simulink model "wlan_phy_rx_pmd"  (Simulink 7.8)
settings: Solver=VariableStepDiscrete  SolverMode=SingleTasking  StartTime=0.0  StopTime=rx_sim_time  RelTol=1e-3
PostLoadFcn:
  | wlan_phy_rx_init
InitFcn:
  | wlan_phy_rx_init
StartFcn:
  | wlan_phy_rx_init
values from neighbouring files (symbols this model uses):
  barker_seq20 = 0.95 * circshift(barker_seq20, [0 4]) ./ max(abs(barker_seq20))    [wlan_phy_rx_init.m]
  bit_scrambler_lfsr_bytes = sum(bit_scrambler_lfsr_bytes .* repmat(2.^[0:7], 127, 1),2)    [wlan_phy_rx_init.m]
  deinterleave_ROM = [deinterleave_ROM interleave_11n_64QAM zeros(1, 512-length(interleave_11n_64QAM))]    [wlan_phy_rx_init.m]
  longCorr_coef_i = [3*real(longCorr_coef)]    [wlan_phy_rx_init.m]
  longCorr_coef_q = [3*imag(longCorr_coef)]    [wlan_phy_rx_init.m]
  MAX_CP_LEN = 32    [wlan_phy_rx_init.m]
  MAX_NCBPS = NCBPS_11n_64QAM    (= 312)    [wlan_phy_rx_init.m]
  MAX_NUM_BYTES = 2^16-1    (= 65535)    [wlan_phy_rx_init.m]
  MAX_NUM_SC = 64    [wlan_phy_rx_init.m]
  MAX_NUM_SYMS = 600    [wlan_phy_rx_init.m]
  NCBPS_11a_BPSK = 48    [wlan_phy_rx_init.m]
  NDBPS_11a_BPSK12 = 24    [wlan_phy_rx_init.m]
  PHY_SIGNAL_MIN_LEN = 14    [wlan_phy_rx_init.m]
  preFFT_sampBuff_numSamps = 4*MAX_NUM_SC    (= 256)    [wlan_phy_rx_init.m]
  rx_sim_sig_samp_time = 8    [wlan_phy_rx_init.m]
  scr_ind_rev = zeros(1,128)    [wlan_phy_rx_init.m]
undefined symbols (not defined in the file or in its folder): gcbh, mcs_rom_11ag, mcs_rom_11n, xlBlockLoadCallback, xlGetNormalizedPeriod, xlipgetpartsetting
libraries: xbsIndex_r4 x3862, simulink x1, xbsEDKLib_r4 x1
(part 7 of 23)

== system wlan_phy_rx_pmd/Sync & Antenna Sel/Antenna Selection ==
  Pkt Det : Inport
  Rx I Vec : Inport  Port=2
  Rx Q Vec : Inport  Port=3
  IQ Valid : Inport  Port=4
  RSSI Vec : Inport  Port=5
  Reset : Inport  Port=6
  Ant Sel Logic : Subsystem
  Bus Selector1 : BusSelector  OutputSignals=RFA_Q,RFB_Q,RFC_Q,RFD_Q
  Bus Selector2 : BusSelector  OutputSignals=RFA_RSSI,RFB_RSSI,RFC_RSSI,RFD_RSSI
  Bus Selector3 : BusSelector  OutputSignals=RFA_I,RFB_I,RFC_I,RFD_I
  Bus Selector4 : BusSelector  OutputSignals=signal1,signal2,signal3,signal4
  Delay : xbsIndex_r4/Delay  rst=off  infoeditControl=Selection of Reset will increase slice count due to use of real FFs and instead of SRLs  en=off  latency=1  dbl_ovrd=off  reg_retiming=off  block_type=delay
  Gateway Out{,1..2,4..6} (6 alike) : xbsIndex_r4/Gateway Out  inherit_from_input=off  hdl_port=off  timing_constraint=None  locs_specified=off  LOCs={}  UseAsDAC=off  DACChannel='1'  block_type=gatewayout
  Logical : xbsIndex_r4/Logical  logical_function=AND  inputs=2  en=off  latency=0  precision=Full  arith_type=Unsigned  n_bits=16  bin_pt=0  align_bp=on  dbl_ovrd=off  block_type=logical
  Mux{1..4} (4 alike) : xbsIndex_r4/Mux  inputs=4  en=off  latency=0  precision=Full  arith_type=Unsigned  n_bits=16  bin_pt=14  quantization=Truncate  overflow=Wrap  dbl_ovrd=off  block_type=mux
  Posedge : Subsystem
  RF A Gains : Subsystem
  RF B Gains : Subsystem
  RF C Gains : Subsystem
  RF D Gains : Subsystem
  RSSI & Gain Capture : Subsystem
  Register3 : xbsIndex_r4/Register  init=0  rst=off  en=on  dbl_ovrd=off  block_type=register
      settings: xl_area=[1 1 0 0 0 0 0]  block_version=VER_STRING_GOES_HERE
  Sel Div : Scope  NumInputPorts=10
      settings: TimeRange=16708  YMin=0~-1~-1~0~-1~-1~-1~-1~-1~-1  YMax=1~1~1~1~1~1~1~1~1~1  SaveName=ScopeData42
  Rx I : Outport
  " Rx Q" : Outport  Port=2
  " IQ Valid" : Outport  Port=3
  RSSI : Outport  Port=4
  tag scope: ANTENNA_SEL=global
  wires:
  RF A Gains/1(G_vec) -> Gateway Out2/1, Ant Sel Logic/1(G A)
  RF B Gains/1(G_vec) -> Gateway Out4/1, Ant Sel Logic/2(G B)
  Rx Q Vec/1 -> Bus Selector1/1
  Reset/1 -> RF B Gains/2(Rst), RF A Gains/2(Rst), RF C Gains/2(Rst), RF D Gains/2(Rst)
  Logical/1 -> Posedge/1(D)
  Pkt Det/1 -> Logical/1, Gateway Out/1
  [AGC_DONE] -> Logical/2, Gateway Out1/1
  Posedge/1(Q) -> RF A Gains/1(Capt), RF B Gains/1(Capt), RF C Gains/1(Capt), Delay/1, RF D Gains/1(Capt)
  Delay/1 -> Register3/2
  Register3/1 -> [ANTENNA_SEL]
  IQ Valid/1 -> Bus Selector4/1
  Bus Selector4/1 -> Mux3/2  "<signal1>"
  Bus Selector4/2 -> Mux3/3  "<signal2>"
  Mux3/1 -> " IQ Valid"/1
  Gateway Out2/1 -> Sel Div/3  "G A"
  Gateway Out1/1 -> Sel Div/2  "AGC Done"
  Gateway Out/1 -> Sel Div/1  "Pkt Det"
  Gateway Out4/1 -> Sel Div/4  "G B"
  RF C Gains/1(G_vec) -> Ant Sel Logic/3(G C), Gateway Out5/1
  RF D Gains/1(G_vec) -> Ant Sel Logic/4(G D), Gateway Out6/1
  Gateway Out5/1 -> Sel Div/5  "G C"
  Gateway Out6/1 -> Sel Div/6  "G D"
  Bus Selector4/3 -> Mux3/4  "<signal3>"
  Bus Selector4/4 -> Mux3/5  "<signal4>"
  Rx I Vec/1 -> Bus Selector3/1
  Bus Selector3/1 -> Mux1/2  "<RFA_I>"
  Bus Selector3/2 -> Mux1/3  "<RFB_I>"
  Bus Selector3/3 -> Mux1/4  "<RFC_I>"
  Bus Selector3/4 -> Mux1/5  "<RFD_I>"
  Bus Selector1/1 -> Mux2/2  "<RFA_Q>"
  Bus Selector1/2 -> Mux2/3  "<RFB_Q>"
  Bus Selector1/3 -> Mux2/4  "<RFC_Q>"
  Bus Selector1/4 -> Mux2/5  "<RFD_Q>"
  RSSI Vec/1 -> Bus Selector2/1, RSSI & Gain Capture/1(RSSI Vec)
  Bus Selector2/1 -> Mux4/2  "<RFA_RSSI>"
  Bus Selector2/2 -> Mux4/3  "<RFB_RSSI>"
  Bus Selector2/3 -> Mux4/4  "<RFC_RSSI>"
  Bus Selector2/4 -> Mux4/5  "<RFD_RSSI>"
  Mux4/1 -> RSSI/1
  Ant Sel Logic/1(Ant Sel) -> Mux3/1, Mux1/1, Mux2/1, Mux4/1, Register3/1
  Mux2/1 -> " Rx Q"/1
  Mux1/1 -> Rx I/1

== system wlan_phy_rx_pmd/Sync & Antenna Sel/Debug  Outputs ==
  note: Pulse stretch the timeout signal
      | so it's visible on debug outputs
      | and in down-sampled CS ILA
  LTS : Inport
  LTS Timeout : Inport  Port=2
  Det OFDM : Inport  Port=3
  Det DSSS : Inport  Port=4
  LTS_SYNC : xbsIndex_r4/Gateway Out  inherit_from_input=off  hdl_port=on  timing_constraint=None  locs_specified=off  LOCs={}  UseAsDAC=off  DACChannel='1'  block_type=gatewayout
  Pulse Stretch : Subsystem
  Pulse Stretch1 : Subsystem
  Register{2..11} (10 alike) : xbsIndex_r4/Register  init=0  rst=off  en=off  dbl_ovrd=off  block_type=register
  Slice2 : xbsIndex_r4/Slice  nbits=1  boolean_output=on  mode=Upper Bit Location + Width  bit1=0  base1=MSB of Input  bit0=0  base0=LSB of Input  dbl_ovrd=off  block_type=slice
  dbg_PKT_DET_DSSS : xbsIndex_r4/Gateway Out  inherit_from_input=off  hdl_port=on  timing_constraint=None  locs_specified=off  LOCs={}  UseAsDAC=off  DACChannel='1'  block_type=gatewayout
  dbg_PKT_DET_OFDM : xbsIndex_r4/Gateway Out  inherit_from_input=off  hdl_port=on  timing_constraint=None  locs_specified=off  LOCs={}  UseAsDAC=off  DACChannel='1'  block_type=gatewayout
  dbg_lts_timeout : xbsIndex_r4/Gateway Out  inherit_from_input=off  hdl_port=on  timing_constraint=None  locs_specified=off  LOCs={}  UseAsDAC=off  DACChannel='1'  block_type=gatewayout
  tag scope: CS_LTS_Timeout=global, CS_Pktdet_DSSS=global, CS_Pktdet_OFDM=global
  wires:
  Register8/1 -> Register9/1
  Register9/1 -> LTS_SYNC/1
  Slice2/1 -> Pulse Stretch/1(P)
  Register2/1 -> Register3/1
  Register3/1 -> dbg_lts_timeout/1
  LTS/1 -> Slice2/1
  Register6/1 -> Register4/1, [CS_Pktdet_OFDM]
  Register7/1 -> dbg_PKT_DET_OFDM/1
  Register4/1 -> Register7/1
  Register10/1 -> Register5/1, [CS_Pktdet_DSSS]
  Register11/1 -> dbg_PKT_DET_DSSS/1
  Register5/1 -> Register11/1
  Det OFDM/1 -> Register6/1
  Det DSSS/1 -> Register10/1
  Pulse Stretch/1(P16) -> Register8/1
  LTS Timeout/1 -> Pulse Stretch1/1(P)
  Pulse Stretch1/1(P16) -> Register2/1, [CS_LTS_Timeout]

== system wlan_phy_rx_pmd/Sync & Antenna Sel/LTS Correlation ==
  rx_I : Inport
  rx_Q : Inport  Port=2
  rx_IQ_valid : Inport  Port=3
  RSSI : Inport  Port=4
  En : Inport  Port=5
  Reset : Inport  Port=6
  Bus Creator : BusCreator  Inputs=2
  Bus Creator1 : BusCreator  Inputs=2
  Concat : xbsIndex_r4/Concat  num_inputs=2  dbl_ovrd=off  block_type=concat
  Correlator : Subsystem
  Gateway Out{1..10} (10 alike) : xbsIndex_r4/Gateway Out  inherit_from_input=off  hdl_port=off  timing_constraint=None  locs_specified=off  LOCs={}  UseAsDAC=off  DACChannel='1'  block_type=gatewayout
  Inverter : xbsIndex_r4/Inverter  en=off  latency=0  dbl_ovrd=off  block_type=inv
      settings: xl_area=[0 0 0 0 0 0 0]  block_version=VER_STRING_GOES_HERE
  Inverter1 : xbsIndex_r4/Inverter  en=off  latency=0  dbl_ovrd=off  block_type=inv
      settings: xl_area=[0 0 0 0 0 0 0]  block_version=VER_STRING_GOES_HERE
  LTS Corr : Scope  NumInputPorts=8
      settings: TimeRange=15000  YMin=-1~-1~0~-1~-1~0~-1~-1  YMax=1~1~15000~1~1~1~1~1  SaveName=ScopeData2
  LTS Corr Timeout : Subsystem
  Logical1 : xbsIndex_r4/Logical  logical_function=AND  inputs=2  en=off  latency=0  precision=Full  arith_type=Unsigned  n_bits=16  bin_pt=0  align_bp=on  dbl_ovrd=off  block_type=logical
  Logical2 : xbsIndex_r4/Logical  logical_function=AND  inputs=3  en=off  latency=0  precision=Full  arith_type=Unsigned  n_bits=16  bin_pt=0  align_bp=on  dbl_ovrd=off  block_type=logical
  Logical3 : xbsIndex_r4/Logical  logical_function=AND  inputs=2  en=off  latency=1  precision=Full  arith_type=Unsigned  n_bits=16  bin_pt=0  align_bp=on  dbl_ovrd=off  block_type=logical
  Posedge3 : Subsystem
  Relational2 : xbsIndex_r4/Relational  mode=a>b  en=off  latency=0  dbl_ovrd=off  block_type=relational
      settings: xl_area=[10 0 0 19 0 0 0]  block_version=VER_STRING_GOES_HERE
  S-R Latch : Subsystem
  S-R Latch1 : Subsystem
  Slice : xbsIndex_r4/Slice  nbits=1  boolean_output=off  mode=Upper Bit Location + Width  bit1=0  base1=MSB of Input  bit0=0  base0=LSB of Input  dbl_ovrd=off  block_type=slice
      settings: infoedit="Extracts a given range of bits from each input sample and presents it at the output.  The output type is ordinarily unsigned with binary point at zero, but can be Boolean when the slice is one bit wide.<P><P>Hardware notes: In hardware this block costs nothing."  block_version=10.1.3
  Slice1 : xbsIndex_r4/Slice  nbits=1  boolean_output=off  mode=Upper Bit Location + Width  bit1=0  base1=MSB of Input  bit0=0  base0=LSB of Input  dbl_ovrd=off  block_type=slice
      settings: infoedit="Extracts a given range of bits from each input sample and presents it at the output.  The output type is ordinarily unsigned with binary point at zero, but can be Boolean when the slice is one bit wide.<P><P>Hardware notes: In hardware this block costs nothing."  block_version=10.1.3
  Threshold Select : Subsystem
  Det : Outport
  Timeout : Outport  Port=2
  tag scope: CS_LTS_Det_1=global, CS_LTS_Det_2=global
  wires:
  Gateway Out2/1 -> LTS Corr/3
  Gateway Out4/1 -> LTS Corr/2
  Logical1/1 -> LTS Corr Timeout/3(LTS 1), Concat/2, [CS_LTS_Det_1], Gateway Out8/1
  Inverter/1 -> Logical1/2
  Reset/1 -> S-R Latch/2(R), S-R Latch1/2(R), Gateway Out10/1
  Logical2/1 -> S-R Latch1/1(S), LTS Corr Timeout/4(LTS 2), Concat/1, [CS_LTS_Det_2], Gateway Out9/1
  S-R Latch/1(Q) -> Inverter/1, Logical2/3
  Gateway Out1/1 -> LTS Corr/4
  Gateway Out3/1 -> LTS Corr/5
  rx_I/1 -> Slice/1, Gateway Out4/1
  rx_Q/1 -> Slice1/1, Gateway Out2/1
  Correlator/1(R) -> Relational2/1, Gateway Out1/1
  Gateway Out7/1 -> LTS Corr/8
  Posedge3/1(Q) -> Logical1/1, Logical2/2, S-R Latch/1(S), Gateway Out3/1
  Logical3/1 -> Posedge3/1(D)
  Relational2/1 -> Logical3/2
  En/1 -> LTS Corr Timeout/1(Pkt Det), Logical3/1, Gateway Out6/1, Threshold Select/1(Pkt Det), Gateway Out5/1
  S-R Latch1/1(Q) -> Inverter1/1
  Inverter1/1 -> Logical2/1
  LTS Corr Timeout/1(Timeout) -> Timeout/1, Gateway Out7/1
  Slice/1 -> Correlator/1(Rx I)
  Slice1/1 -> Correlator/2(Rx Q)
  rx_IQ_valid/1 -> LTS Corr Timeout/2(IQ Valid), Correlator/3(rx_IQ_valid)
  Bus Creator/1 -> LTS Corr/6
  Bus Creator1/1 -> LTS Corr/7
  Gateway Out8/1 -> Bus Creator/1
  Gateway Out9/1 -> Bus Creator/2
  Gateway Out6/1 -> Bus Creator1/1
  Gateway Out10/1 -> Bus Creator1/2
  Threshold Select/1(Corr Thresh) -> Relational2/2
  RSSI/1 -> Threshold Select/2(RSSI)
  Concat/1 -> Det/1
  Gateway Out5/1 -> LTS Corr/1

== system wlan_phy_rx_pmd/Sync & Antenna Sel/PHY CCA ==
  LTS Sync : Inport
  LTS Timeout : Inport  Port=2
  IQ Valid : Inport  Port=3
  Det OFDM : Inport  Port=4
  Avg RSSI Vec : Inport  Port=5
  Reset OFDM : Inport  Port=6
  Det DSSS : Inport  Port=7
  Reset DSSS : Inport  Port=8
  Bus Selector1 : BusSelector  OutputSignals=RFA_RSSI,RFB_RSSI,RFC_RSSI,RFD_RSSI
  Gateway Out1 : xbsIndex_r4/Gateway Out  inherit_from_input=off  hdl_port=off  timing_constraint=None  locs_specified=off  LOCs={}  UseAsDAC=off  DACChannel='1'  block_type=gatewayout
  Gateway Out2 : xbsIndex_r4/Gateway Out  inherit_from_input=off  hdl_port=off  timing_constraint=None  locs_specified=off  LOCs={}  UseAsDAC=off  DACChannel='1'  block_type=gatewayout
  Gateway Out4 : xbsIndex_r4/Gateway Out  inherit_from_input=off  hdl_port=off  timing_constraint=None  locs_specified=off  LOCs={}  UseAsDAC=off  DACChannel='1'  block_type=gatewayout
  Logical1 : xbsIndex_r4/Logical  logical_function=OR  inputs=2  en=off  latency=0  precision=Full  arith_type=Unsigned  n_bits=16  bin_pt=0  align_bp=on  dbl_ovrd=off  block_type=logical
  Logical2 : xbsIndex_r4/Logical  logical_function=OR  inputs=4  en=off  latency=0  precision=Full  arith_type=Unsigned  n_bits=16  bin_pt=0  align_bp=on  dbl_ovrd=off  block_type=logical
  Logical3 : xbsIndex_r4/Logical  logical_function=AND  inputs=2  en=off  latency=0  precision=Full  arith_type=Unsigned  n_bits=16  bin_pt=0  align_bp=on  dbl_ovrd=off  block_type=logical
  Logical4 : xbsIndex_r4/Logical  logical_function=AND  inputs=2  en=off  latency=0  precision=Full  arith_type=Unsigned  n_bits=16  bin_pt=0  align_bp=on  dbl_ovrd=off  block_type=logical
  PHY CCA : Scope  NumInputPorts=7
      settings: TimeRange=10000  YMin=-1~-1~0.95~-70~0~0~-0.25  YMax=1~1~1.05~80~1~60000~0.3  SaveName=ScopeData31
  PHY CCA Logic : Subsystem
  Rate*Length BUSY : Subsystem
  Relational{,1..3} (4 alike) : xbsIndex_r4/Relational  mode=a>b  en=off  latency=0  dbl_ovrd=off  block_type=relational
  S-R Latch1 : Subsystem
  S-R Latch2 : Subsystem
  S-R Latch3 : Subsystem
  Slice2 : xbsIndex_r4/Slice  nbits=1  boolean_output=on  mode=Upper Bit Location + Width  bit1=0  base1=MSB of Input  bit0=0  base0=LSB of Input  dbl_ovrd=off  block_type=slice
  tag scope: RX_CCA_BUSY=global, RX_END_OFDM_LAST_SAMP=global, RX_PHY_RATE_LENGTH_ACTIVE=global
  wires:
  [regRx_RSSI_CS_THRESH] -> Relational/2, Relational1/2, Relational2/2, Relational3/2
  Bus Selector1/1 -> Relational/1  "<RFA_RSSI>"
  Logical2/1 -> [RX_CCA_BUSY], Gateway Out2/1  "CCA_BUSY"
  Det OFDM/1 -> S-R Latch2/1(S)
  Logical1/1 -> S-R Latch2/2(R)
  Reset OFDM/1 -> Logical1/1
  LTS Timeout/1 -> Logical1/2
  S-R Latch2/1(Q) -> Logical3/2, Logical2/3
  IQ Valid/1 -> Rate*Length BUSY/1(IQ Valid)
  Logical3/1 -> Rate*Length BUSY/2(Start), Gateway Out4/1
  LTS Sync/1 -> Slice2/1
  Avg RSSI Vec/1 -> Bus Selector1/1
  Slice2/1 -> Logical3/1
  Relational/1 -> PHY CCA Logic/1(Det A)
  Relational1/1 -> PHY CCA Logic/2(Det B)
  Bus Selector1/2 -> Relational1/1  "<RFB_RSSI>"
  PHY CCA Logic/1(BUSY) -> Logical2/2
  Bus Selector1/3 -> Relational2/1  "<RFC_RSSI>"
  Bus Selector1/4 -> Relational3/1  "<RFD_RSSI>"
  Relational2/1 -> PHY CCA Logic/3(Det C)
  Relational3/1 -> PHY CCA Logic/4(Det D)
  Gateway Out4/1 -> PHY CCA/1  "Start"
  Gateway Out1/1 -> PHY CCA/2  "RL Active"
  Gateway Out2/1 -> PHY CCA/3  "CCA Busy"
  [OFDM_RX_SIGNAL_VALID] -> S-R Latch1/1(S)
  S-R Latch1/1(Q) -> Logical2/1, [RX_PHY_RATE_LENGTH_ACTIVE], Gateway Out1/1
  Rate*Length BUSY/1(Pkt Done) -> S-R Latch1/2(R), [RX_END_OFDM_LAST_SAMP]
  Det DSSS/1 -> S-R Latch3/1(S)
  Reset DSSS/1 -> S-R Latch3/2(R)
  [regRx_DSSS_asserts_CCA_busy] -> Logical4/1
  S-R Latch3/1(Q) -> Logical4/2
  Logical4/1 -> Logical2/4

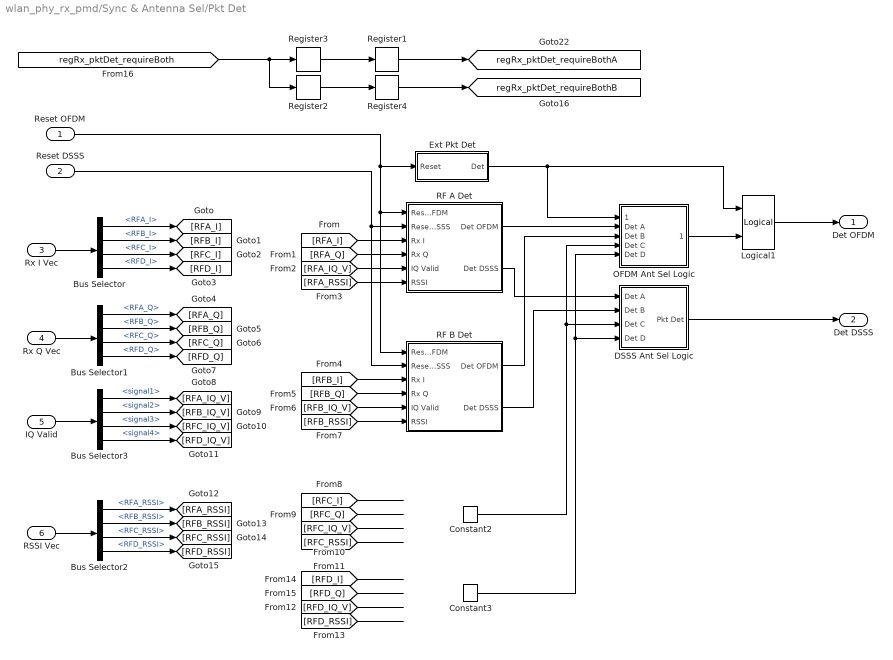
== system wlan_phy_rx_pmd/Sync & Antenna Sel/Pkt Det ==
  Reset OFDM : Inport
  Reset DSSS : Inport  Port=2
  Rx I Vec : Inport  Port=3
  Rx Q Vec : Inport  Port=4
  IQ Valid : Inport  Port=5
  RSSI Vec : Inport  Port=6
  Bus Selector : BusSelector  OutputSignals=RFA_I,RFB_I,RFC_I,RFD_I
  Bus Selector1 : BusSelector  OutputSignals=RFA_Q,RFB_Q,RFC_Q,RFD_Q
  Bus Selector2 : BusSelector  OutputSignals=RFA_RSSI,RFB_RSSI,RFC_RSSI,RFD_RSSI
  Bus Selector3 : BusSelector  OutputSignals=signal1,signal2,signal3,signal4
  Constant2 : xbsIndex_r4/Constant  const=0  gui_display_data_type=Boolean  arith_type=Boolean  n_bits=1  bin_pt=0  preci_type=Single  exp_width=8  frac_width=24  explicit_period=off  period=1  equ=P=C  opselect=C  inp2=PCIN>>17  opr=+  inp1=P  carry=CIN  dbl_ovrd=off  block_type=constant
      settings: block_version=VER_STRING_GOES_HERE
  Constant3 : xbsIndex_r4/Constant  const=0  gui_display_data_type=Boolean  arith_type=Boolean  n_bits=1  bin_pt=0  preci_type=Single  exp_width=8  frac_width=24  explicit_period=off  period=1  equ=P=C  opselect=C  inp2=PCIN>>17  opr=+  inp1=P  carry=CIN  dbl_ovrd=off  block_type=constant
      settings: block_version=VER_STRING_GOES_HERE
  DSSS Ant Sel Logic : Subsystem
  Ext Pkt Det : Subsystem
  Logical1 : xbsIndex_r4/Logical  logical_function=OR  inputs=2  en=off  latency=0  precision=Full  arith_type=Unsigned  n_bits=16  bin_pt=0  align_bp=on  dbl_ovrd=off  block_type=logical
  OFDM Ant Sel Logic : Subsystem
  RF A Det : Subsystem
  RF B Det : Subsystem
  Register{1..4} (4 alike) : xbsIndex_r4/Register  init=0  rst=off  en=off  dbl_ovrd=off  block_type=register
  Det OFDM : Outport
  Det DSSS : Outport  Port=2
  tag scope: regRx_pktDet_requireBothA=global, regRx_pktDet_requireBothB=global
  wires:
  Rx Q Vec/1 -> Bus Selector1/1
  IQ Valid/1 -> Bus Selector3/1
  RSSI Vec/1 -> Bus Selector2/1
  RF A Det/1(Det OFDM) -> OFDM Ant Sel Logic/2(Det A)
  OFDM Ant Sel Logic/1(Pkt Det) -> Logical1/2
  Rx I Vec/1 -> Bus Selector/1
  Logical1/1 -> Det OFDM/1
  Ext Pkt Det/1(Det) -> Logical1/1, OFDM Ant Sel Logic/1(Ext Det)
  Bus Selector/1 -> [RFA_I]  "<RFA_I>"
  Bus Selector/2 -> [RFB_I]  "<RFB_I>"
  Bus Selector/3 -> [RFC_I]  "<RFC_I>"
  Bus Selector/4 -> [RFD_I]  "<RFD_I>"
  Bus Selector1/1 -> [RFA_Q]  "<RFA_Q>"
  Bus Selector1/2 -> [RFB_Q]  "<RFB_Q>"
  Bus Selector1/3 -> [RFC_Q]  "<RFC_Q>"
  Bus Selector1/4 -> [RFD_Q]  "<RFD_Q>"
  Bus Selector3/1 -> [RFA_IQ_V]  "<signal1>"
  Bus Selector3/2 -> [RFB_IQ_V]  "<signal2>"
  Bus Selector3/3 -> [RFC_IQ_V]  "<signal3>"
  Bus Selector3/4 -> [RFD_IQ_V]  "<signal4>"
  Bus Selector2/1 -> [RFA_RSSI]  "<RFA_RSSI>"
  Bus Selector2/2 -> [RFB_RSSI]  "<RFB_RSSI>"
  Bus Selector2/3 -> [RFC_RSSI]  "<RFC_RSSI>"
  Bus Selector2/4 -> [RFD_RSSI]  "<RFD_RSSI>"
  [RFA_I] -> RF A Det/3(Rx I)
  [RFA_Q] -> RF A Det/4(Rx Q)
  [RFA_IQ_V] -> RF A Det/5(IQ Valid)
  [RFA_RSSI] -> RF A Det/6(RSSI)
  [RFB_I] -> RF B Det/3(Rx I)
  [RFB_Q] -> RF B Det/4(Rx Q)
  [RFB_IQ_V] -> RF B Det/5(IQ Valid)
  [RFB_RSSI] -> RF B Det/6(RSSI)
  [RFC_I] -> ?
  [RFC_Q] -> ?
  [RFC_IQ_V] -> ?
  [RFC_RSSI] -> ?
  [RFD_I] -> ?
  [RFD_Q] -> ?
  [RFD_IQ_V] -> ?
  [RFD_RSSI] -> ?
  RF A Det/2(Det DSSS) -> DSSS Ant Sel Logic/1(Det A)
  DSSS Ant Sel Logic/1(Pkt Det) -> Det DSSS/1
  Reset OFDM/1 -> Ext Pkt Det/1(Reset), RF A Det/1(Reset OFDM), RF B Det/1(Reset OFDM)
  Reset DSSS/1 -> RF A Det/2(Reset DSSS), RF B Det/2(Reset DSSS)
  Constant2/1 -> OFDM Ant Sel Logic/4(Det C), DSSS Ant Sel Logic/3(Det C)
  Constant3/1 -> OFDM Ant Sel Logic/5(Det D), DSSS Ant Sel Logic/4(Det D)
  RF B Det/1(Det OFDM) -> OFDM Ant Sel Logic/3(Det B)
  RF B Det/2(Det DSSS) -> DSSS Ant Sel Logic/2(Det B)
  Register3/1 -> Register1/1
  [regRx_pktDet_requireBoth] -> Register3/1, Register2/1
  Register2/1 -> Register4/1
  Register1/1 -> [regRx_pktDet_requireBothA]
  Register4/1 -> [regRx_pktDet_requireBothB]

== system wlan_phy_rx_pmd/Sync & Antenna Sel/Pkt Det AGC Ctrl ==
  note: Pkt det output to start/reset AGC
  Det OFDM : Inport
  Det DSSS : Inport  Port=2
  AGC Ctrl : Scope  NumInputPorts=4
      settings: YMin=-5~-5~-5~-5  YMax=5~5~5~5  SaveName=ScopeData24
  Gateway Out : xbsIndex_r4/Gateway Out  inherit_from_input=off  hdl_port=off  timing_constraint=None  locs_specified=off  LOCs={}  UseAsDAC=off  DACChannel='1'  block_type=gatewayout
  Gateway Out1 : xbsIndex_r4/Gateway Out  inherit_from_input=off  hdl_port=off  timing_constraint=None  locs_specified=off  LOCs={}  UseAsDAC=off  DACChannel='1'  block_type=gatewayout
  Logical : xbsIndex_r4/Logical  logical_function=OR  inputs=2  en=off  latency=0  precision=Full  arith_type=Unsigned  n_bits=16  bin_pt=0  align_bp=on  dbl_ovrd=off  block_type=logical
  Register1 : xbsIndex_r4/Register  init=0  rst=off  en=off  dbl_ovrd=off  block_type=register
  pkt_det_o : xbsIndex_r4/Gateway Out  inherit_from_input=off  hdl_port=on  timing_constraint=None  locs_specified=off  LOCs={}  UseAsDAC=off  DACChannel='1'  block_type=gatewayout
  wires:
  Register1/1 -> pkt_det_o/1
  Logical/1 -> Register1/1
  Det OFDM/1 -> Logical/1, Gateway Out/1
  Gateway Out/1 -> AGC Ctrl/1
  Gateway Out1/1 -> AGC Ctrl/2
  Det DSSS/1 -> Logical/2, Gateway Out1/1

== system wlan_phy_rx_pmd/Sync & Antenna Sel/Resets ==
  note: Handle rare case of a valid SIGNAL being received, sending
      | RX_START to MAC, then getting reset before the pkt is processed.
      | RX_START must be followed by RX_END for sane MAC state.
  OFDM Reset : Inport
  "DSSS Reset " : Inport  Port=2
  LTS Timeout : Inport  Port=3
  Constant1 : Constant  Value=0
  Inverter4 : xbsIndex_r4/Inverter  en=off  latency=0  dbl_ovrd=off  block_type=inv
  Logical1 : xbsIndex_r4/Logical  logical_function=AND  inputs=2  en=off  latency=0  precision=Full  arith_type=Unsigned  n_bits=16  bin_pt=0  align_bp=on  dbl_ovrd=off  block_type=logical
  Logical2 : xbsIndex_r4/Logical  logical_function=OR  inputs=4  en=off  latency=0  precision=Full  arith_type=Unsigned  n_bits=16  bin_pt=0  align_bp=on  dbl_ovrd=off  block_type=logical
  Logical3 : xbsIndex_r4/Logical  logical_function=OR  inputs=2  en=off  latency=0  precision=Full  arith_type=Unsigned  n_bits=16  bin_pt=0  align_bp=on  dbl_ovrd=off  block_type=logical
  Logical4 : xbsIndex_r4/Logical  logical_function=OR  inputs=3  en=off  latency=0  precision=Full  arith_type=Unsigned  n_bits=16  bin_pt=0  align_bp=on  dbl_ovrd=off  block_type=logical
  Logical5 : xbsIndex_r4/Logical  logical_function=OR  inputs=2  en=off  latency=0  precision=Full  arith_type=Unsigned  n_bits=16  bin_pt=0  align_bp=on  dbl_ovrd=off  block_type=logical
  Logical6 : xbsIndex_r4/Logical  logical_function=AND  inputs=2  en=off  latency=0  precision=Full  arith_type=Unsigned  n_bits=16  bin_pt=0  align_bp=on  dbl_ovrd=off  block_type=logical
  Mux : xbsIndex_r4/Mux  inputs=2  en=off  latency=0  precision=Full  arith_type=Unsigned  n_bits=16  bin_pt=14  quantization=Truncate  overflow=Wrap  dbl_ovrd=off  block_type=mux
  Negedge : Subsystem
  PHY_RX_BLOCK_PKTDET : xbsIndex_r4/Gateway In  gui_display_data_type=Boolean  arith_type=Boolean  n_bits=16  bin_pt=14  preci_type=Single  exp_width=8  frac_width=24  quantization="Round  (unbiased: +/- Inf)"  overflow=Saturate  period=1  dbl_ovrd=off  timing_constraint=None  locs_specified=off  LOCs={}  UseAsADC=off  ADCChannel='1'  inherit_from_input=off  hdl_port=on  block_type=gatewayin
  Register3 : xbsIndex_r4/Register  init=0  rst=off  en=off  dbl_ovrd=off  block_type=register
  Reset Gen : Subsystem
  Reset Gen1 : Subsystem
  " OFDM Reset" : Outport
  DSSS Reset : Outport  Port=2
  tag scope: PKT_DET_BLOCK=global, RX_ENDED_UNEXPECTEDLY=global
  wires:
  Logical1/1 -> [RX_ENDED_UNEXPECTEDLY]
  Logical3/1 -> Logical1/1
  [RX_STARTED_TO_MAC] -> Logical1/2
  Register3/1 -> [PKT_DET_BLOCK], Logical3/1, Logical2/4, Logical4/3
  PHY_RX_BLOCK_PKTDET/1 -> Register3/1
  [RX_GLOBAL_RESET] -> Logical3/2, Logical2/3, Logical4/2
  Logical2/1 -> Reset Gen/1(Done)
  Reset Gen/1(Reset) -> " OFDM Reset"/1
  Constant1/1 -> PHY_RX_BLOCK_PKTDET/1
  LTS Timeout/1 -> Logical2/2
  Reset Gen1/1(Reset) -> DSSS Reset/1
  Logical4/1 -> Reset Gen1/1(Done)
  "DSSS Reset "/1 -> Logical4/1
  [RX_PHY_RATE_LENGTH_ACTIVE] -> Inverter4/1, Negedge/1(D)
  Inverter4/1 -> Logical6/2
  Logical6/1 -> Logical5/1
  Negedge/1(Q) -> Logical5/2
  OFDM Reset/1 -> Logical6/1, Mux/2
  Logical5/1 -> Mux/3
  Mux/1 -> Logical2/1
  [regRx_rateLength_holds_pktDet] -> Mux/1

== system wlan_phy_rx_pmd/CFO & Samp Buffer/CFO Correction/Sim Reset ==
  Constant4 : xbsIndex_r4/Constant  const=0  gui_display_data_type=Boolean  arith_type=Boolean  n_bits=16  bin_pt=14  preci_type=Single  exp_width=8  frac_width=24  explicit_period=on  period=1  equ=P=C  opselect=C  inp2=PCIN>>17  opr=+  inp1=P  carry=CIN  dbl_ovrd=off  block_type=constant
  Simulation Multiplexer : xbsIndex_r4/Simulation Multiplexer  sim_sel=1  hw_sel=2  block_type=simmux
      settings: infoedit="Distinguish input subsystems as \"simulation only\" and \"generation only\".  The input specified For Simulation will be used during Simulink simulation.  The input specified For Generation will be used during code generation.  This block will typically be used on the output of a HW Co-Simulation, Black Box, or ModelSim subsystem.<br><br>Hardware Notes: This block costs nothing."
  Subsystem : Subsystem
  Out1 : Outport
  wires:
  Subsystem/1(Out1) -> Simulation Multiplexer/1
  Constant4/1 -> Simulation Multiplexer/2
  Simulation Multiplexer/1 -> Out1/1

== system wlan_phy_rx_pmd/CFO & Samp Buffer/CFO Estimation/Conj Mult ==
  Valid : Inport
  A I : Inport  Port=2
  A Q : Inport  Port=3
  B I : Inport  Port=4
  B Q : Inport  Port=5
  AddSub : xbsIndex_r4/AddSub  mode=Addition  use_carryin=off  use_carryout=off  en=off  latency=1  precision=User Defined  arith_type="Signed  (2's comp)"  n_bits=17  bin_pt=14  quantization=Truncate  overflow=Flag as error  dbl_ovrd=off  use_behavioral_HDL=off  hw_selection=Fabric  pipelined=off  block_type=addsub
  AddSub1 : xbsIndex_r4/AddSub  mode=Subtraction  use_carryin=off  use_carryout=off  en=off  latency=1  precision=User Defined  arith_type="Signed  (2's comp)"  n_bits=17  bin_pt=14  quantization=Truncate  overflow=Flag as error  dbl_ovrd=off  use_behavioral_HDL=off  hw_selection=Fabric  pipelined=off  block_type=addsub
  Delay : xbsIndex_r4/Delay  rst=off  infoeditControl=Selection of Reset will increase slice count due to use of real FFs and instead of SRLs  en=off  latency=3  dbl_ovrd=off  reg_retiming=off  block_type=delay
  Mult{,1..3} (4 alike) : xbsIndex_r4/Mult  precision=User Defined  arith_type="Signed  (2's comp)"  n_bits=16  bin_pt=14  quantization=Truncate  overflow=Flag as error  en=off  latency=1  dbl_ovrd=off  use_behavioral_HDL=off  opt=Speed  use_embedded=on  optimum_pipeline=off  pipeline=on  use_rpm=on  placement_style=Triangular  block_type=mult
  Register{1..4} (4 alike) : xbsIndex_r4/Register  init=0  rst=off  en=on  dbl_ovrd=off  block_type=register
  " Valid" : Outport
  " I" : Outport  Port=2
  " Q" : Outport  Port=3
  wires:
  B Q/1 -> Register4/1
  Valid/1 -> Delay/1, Register1/2, Register2/2, Register3/2, Register4/2
  A I/1 -> Register1/1
  B I/1 -> Register2/1
  Mult/1 -> AddSub/1
  Mult1/1 -> AddSub/2
  AddSub/1 -> " I"/1
  Delay/1 -> " Valid"/1
  AddSub1/1 -> " Q"/1
  Register1/1 -> Mult/1, Mult2/1
  Register2/1 -> Mult/2, Mult3/1
  Register3/1 -> Mult1/1, Mult3/2
  Register4/1 -> Mult1/2, Mult2/2
  A Q/1 -> Register3/1
  Mult2/1 -> AddSub1/1
  Mult3/1 -> AddSub1/2

== system wlan_phy_rx_pmd/CFO & Samp Buffer/CFO Estimation/Phase ==
  Valid : Inport
  Re : Inport  Port=2
  Im : Inport  Port=3
  "CORDIC 5.0 " : xbsIndex_r4/CORDIC 5.0   functional_selection=Arc_Tan  architectural_configuration=Parallel  pipelining_mode=Optimal  data_format=SignedFraction  phase_format=Scaled_Radians  input_width=18  output_width=18  round_mode=Truncate  iterations=0  precision=0  compensation_scaling=No_Scale_Compensation  coarse_rotation=on  aclken=off  aresetn=off  out_tready=off  cartesian_has_tuser=off  cartesian_has_tlast=off  cartesian_tuser_width=1  phase_has_tuser=off  phase_has_tlast=off  phase_tuser_width=1  out_tlast_behv=Null  flow_control=NonBlocking  optimize_goal=Performance  trim_axipin_name=on  ip_name=CORDIC  ip_version=5.0  dsptool_ready=true  ipcore_usecache=true  ipcore_useipmodelcache=true  wrapper_available=true  port_translation_map={ 'aclken' => 'en', 'aresetn' => 'rst' }  block_type=cordic_v5_0
      settings: infoedit=CORDIC 5.0
  Convert : xbsIndex_r4/Convert  gui_display_data_type=Fixed-point  arith_type="Signed  (2's comp)"  n_bits=18  bin_pt=12  float_type=Single  exp_bits=8  fraction_bits=24  quantization=Truncate  overflow=Wrap  en=off  latency=0  dbl_ovrd=off  pipeline=off  block_type=convert
  Convert1 : xbsIndex_r4/Convert  gui_display_data_type=Fixed-point  arith_type="Signed  (2's comp)"  n_bits=18  bin_pt=12  float_type=Single  exp_bits=8  fraction_bits=24  quantization=Truncate  overflow=Wrap  en=off  latency=0  dbl_ovrd=off  pipeline=off  block_type=convert
  Gateway Out{,1..6} (7 alike) : xbsIndex_r4/Gateway Out  inherit_from_input=off  hdl_port=off  timing_constraint=None  locs_specified=off  LOCs={}  UseAsDAC=off  DACChannel='1'  block_type=gatewayout
  "Phase " : Scope  NumInputPorts=7
      settings: TimeRange=10000  YMin=-2~-5~-2~-5~-0.4~-1~-1  YMax=4~15~4~15~0.4~2~1  SaveName=ScopeData35
  Scale : xbsIndex_r4/Scale  scale_factor=-4  dbl_ovrd=off  block_type=scale
      settings: block_version=10.1.2
  Scale1 : xbsIndex_r4/Scale  scale_factor=-4  dbl_ovrd=off  block_type=scale
      settings: block_version=10.1.2
  " Valid" : Outport
  Phase : Outport  Port=2
  wires:
  Convert1/1 -> Scale1/1, Gateway Out3/1
  Valid/1 -> "CORDIC 5.0 "/1
  "CORDIC 5.0 "/1 -> " Valid"/1
  "CORDIC 5.0 "/2 -> Phase/1, Gateway Out6/1
  Convert/1 -> Scale/1, Gateway Out2/1
  Scale/1 -> "CORDIC 5.0 "/2, Gateway Out4/1
  Scale1/1 -> "CORDIC 5.0 "/3, Gateway Out5/1
  Im/1 -> Convert/1, Gateway Out/1
  Re/1 -> Convert1/1, Gateway Out1/1
  Gateway Out3/1 -> "Phase "/4
  Gateway Out2/1 -> "Phase "/3
  Gateway Out1/1 -> "Phase "/2
  Gateway Out/1 -> "Phase "/1
  Gateway Out4/1 -> "Phase "/5
  Gateway Out5/1 -> "Phase "/6
  Gateway Out6/1 -> "Phase "/7

== system wlan_phy_rx_pmd/CFO & Samp Buffer/CFO Estimation/Posedge3 ==
  note: Rising Edge Detection
  D : Inport
  Delay : xbsIndex_r4/Delay  rst=off  infoeditControl=Selection of Reset will increase slice count due to use of real FFs and instead of SRLs  en=off  latency=1  dbl_ovrd=off  reg_retiming=off  block_type=delay
      settings: infoedit=Hardware notes: A delay line is a chain, each link of which is an SRL16 followed by a flip-flop. If register retiming is enabled, the delay line is a chain of flip-flops.  xl_area=[1 1 0 0 0 0 0]  block_version=VER_STRING_GOES_HERE
  Inverter : xbsIndex_r4/Inverter  en=off  latency=0  dbl_ovrd=off  block_type=inv
      settings: xl_area=[0 0 0 0 0 0 0]  block_version=VER_STRING_GOES_HERE
  Logical1 : xbsIndex_r4/Logical  logical_function=AND  inputs=2  en=off  latency=0  precision=Full  arith_type=Unsigned  n_bits=8  bin_pt=2  align_bp=on  dbl_ovrd=off  block_type=logical
      settings: xl_area=[1 0 0 1 0 0 0]  block_version=VER_STRING_GOES_HERE
  Q : Outport
  wires:
  D/1 -> Logical1/2, Delay/1
  Logical1/1 -> Q/1
  Inverter/1 -> Logical1/1
  Delay/1 -> Inverter/1

== system wlan_phy_rx_pmd/CFO & Samp Buffer/CFO Estimation/Running Sum ==
  En : Inport
  D : Inport  Port=2
  Accum1 : xbsIndex_r4/Accumulator  operation=Add  n_bits=25  overflow=Flag as error  scale=1  rst=off  infoeditControl=reset for floating point data type must be asserted for a minimum of 2 cycles  hasbypass=off  en=on  latency=0  dbl_ovrd=off  msb_inp=100  msb=100  lsb=-100  use_behavioral_HDL=on  implementation=Fabric  block_type=accum
      settings: xl_area=[12 23 0 22 0 0 0]  block_version=VER_STRING_GOES_HERE
  AddSub1 : xbsIndex_r4/AddSub  mode=Subtraction  use_carryin=off  use_carryout=off  en=off  latency=1  precision=Full  arith_type="Signed  (2's comp)"  n_bits=36  bin_pt=28  quantization=Truncate  overflow=Saturate  dbl_ovrd=off  use_behavioral_HDL=on  hw_selection=Fabric  pipelined=off  use_rpm=off  block_type=addsub
      settings: xl_area=[9 18 0 17 0 0 0]  block_version=VER_STRING_GOES_HERE
  Delay : xbsIndex_r4/Delay  rst=off  infoeditControl=Selection of Reset will increase slice count due to use of real FFs and instead of SRLs  en=off  latency=1  dbl_ovrd=off  reg_retiming=off  block_type=delay
      settings: xl_area=[7 14 0 14 0 0 0]  block_version=VER_STRING_GOES_HERE
  Delay1 : xbsIndex_r4/Delay  rst=off  infoeditControl=Selection of Reset will increase slice count due to use of real FFs and instead of SRLs  en=off  latency=1  dbl_ovrd=off  reg_retiming=off  block_type=delay
      settings: xl_area=[7 14 0 14 0 0 0]  block_version=VER_STRING_GOES_HERE
  Delay2 : xbsIndex_r4/Delay  rst=off  infoeditControl=Selection of Reset will increase slice count due to use of real FFs and instead of SRLs  en=off  latency=1  dbl_ovrd=off  reg_retiming=off  block_type=delay
      settings: xl_area=[7 14 0 14 0 0 0]  block_version=VER_STRING_GOES_HERE
  Delay6 : xbsIndex_r4/Delay  rst=off  infoeditControl=Selection of Reset will increase slice count due to use of real FFs and instead of SRLs  en=on  latency=64  dbl_ovrd=off  reg_retiming=off  block_type=delay
      settings: xl_area=[9 17 0 17 0 0 0]  block_version=VER_STRING_GOES_HERE
  Register : xbsIndex_r4/Register  init=0  rst=off  en=off  dbl_ovrd=off  block_type=register
  Valid : Outport
  Sum : Outport  Port=2
  wires:
  Delay6/1 -> AddSub1/2
  AddSub1/1 -> Accum1/1
  D/1 -> AddSub1/1, Delay6/1
  Accum1/1 -> Register/1
  Register/1 -> Sum/1
  En/1 -> Delay6/2, Delay/1
  Delay/1 -> Accum1/2, Delay1/1
  Delay1/1 -> Delay2/1
  Delay2/1 -> Valid/1

== system wlan_phy_rx_pmd/CFO & Samp Buffer/CFO Estimation/Running Sum1 ==
  En : Inport
  D : Inport  Port=2
  Accum1 : xbsIndex_r4/Accumulator  operation=Add  n_bits=25  overflow=Flag as error  scale=1  rst=off  infoeditControl=reset for floating point data type must be asserted for a minimum of 2 cycles  hasbypass=off  en=on  latency=0  dbl_ovrd=off  msb_inp=100  msb=100  lsb=-100  use_behavioral_HDL=on  implementation=Fabric  block_type=accum
      settings: xl_area=[12 23 0 22 0 0 0]  block_version=VER_STRING_GOES_HERE
  AddSub1 : xbsIndex_r4/AddSub  mode=Subtraction  use_carryin=off  use_carryout=off  en=off  latency=1  precision=Full  arith_type="Signed  (2's comp)"  n_bits=36  bin_pt=28  quantization=Truncate  overflow=Saturate  dbl_ovrd=off  use_behavioral_HDL=on  hw_selection=Fabric  pipelined=off  use_rpm=off  block_type=addsub
      settings: xl_area=[9 18 0 17 0 0 0]  block_version=VER_STRING_GOES_HERE
  Delay : xbsIndex_r4/Delay  rst=off  infoeditControl=Selection of Reset will increase slice count due to use of real FFs and instead of SRLs  en=off  latency=1  dbl_ovrd=off  reg_retiming=off  block_type=delay
      settings: xl_area=[7 14 0 14 0 0 0]  block_version=VER_STRING_GOES_HERE
  Delay1 : xbsIndex_r4/Delay  rst=off  infoeditControl=Selection of Reset will increase slice count due to use of real FFs and instead of SRLs  en=off  latency=1  dbl_ovrd=off  reg_retiming=off  block_type=delay
      settings: xl_area=[7 14 0 14 0 0 0]  block_version=VER_STRING_GOES_HERE
  Delay2 : xbsIndex_r4/Delay  rst=off  infoeditControl=Selection of Reset will increase slice count due to use of real FFs and instead of SRLs  en=off  latency=1  dbl_ovrd=off  reg_retiming=off  block_type=delay
      settings: xl_area=[7 14 0 14 0 0 0]  block_version=VER_STRING_GOES_HERE
  Delay6 : xbsIndex_r4/Delay  rst=off  infoeditControl=Selection of Reset will increase slice count due to use of real FFs and instead of SRLs  en=on  latency=64  dbl_ovrd=off  reg_retiming=off  block_type=delay
      settings: xl_area=[9 17 0 17 0 0 0]  block_version=VER_STRING_GOES_HERE
  Register : xbsIndex_r4/Register  init=0  rst=off  en=off  dbl_ovrd=off  block_type=register
  Valid : Outport
  Sum : Outport  Port=2
  wires:
  Delay2/1 -> Valid/1
  Delay1/1 -> Delay2/1
  Delay/1 -> Delay1/1, Accum1/2
  En/1 -> Delay/1, Delay6/2
  Register/1 -> Sum/1
  Accum1/1 -> Register/1
  D/1 -> Delay6/1, AddSub1/1
  AddSub1/1 -> Accum1/1
  Delay6/1 -> AddSub1/2

== system wlan_phy_rx_pmd/CFO & Samp Buffer/CFO Estimation/S-R Latch ==
  S : Inport
  R : Inport  Port=2
  Constant1 : xbsIndex_r4/Constant  const=1  gui_display_data_type=Boolean  arith_type=Boolean  n_bits=1  bin_pt=0  preci_type=Single  exp_width=8  frac_width=24  explicit_period=off  period=1  equ=P=C  opselect=C  inp2=PCIN>>17  opr=+  inp1=P  carry=CIN  dbl_ovrd=off  block_type=constant
      settings: block_version=VER_STRING_GOES_HERE
  Convert1 : xbsIndex_r4/Convert  gui_display_data_type=Boolean  arith_type=Boolean  n_bits=1  bin_pt=0  float_type=Single  exp_bits=8  fraction_bits=24  quantization=Truncate  overflow=Wrap  en=off  latency=0  dbl_ovrd=off  pipeline=off  block_type=convert
  Convert2 : xbsIndex_r4/Convert  gui_display_data_type=Boolean  arith_type=Boolean  n_bits=1  bin_pt=0  float_type=Single  exp_bits=8  fraction_bits=24  quantization=Truncate  overflow=Wrap  en=off  latency=0  dbl_ovrd=off  pipeline=off  block_type=convert
  Register2 : xbsIndex_r4/Register  init=0  rst=on  en=on  dbl_ovrd=off  block_type=register
      settings: xl_area=[1 1 0 0 0 0 0]  block_version=VER_STRING_GOES_HERE
  Q : Outport
  wires:
  Convert2/1 -> Register2/3
  Convert1/1 -> Register2/2
  Constant1/1 -> Register2/1
  R/1 -> Convert1/1
  Register2/1 -> Q/1
  S/1 -> Convert2/1

== system wlan_phy_rx_pmd/CFO & Samp Buffer/FIFO/I/Q Concat ==
  I : Inport
  Q : Inport  Port=2
  Concat : xbsIndex_r4/Concat  num_inputs=2  dbl_ovrd=off  block_type=concat
  Reinterpret : xbsIndex_r4/Reinterpret  force_arith_type=on  arith_type=Unsigned  force_bin_pt=on  bin_pt=0  block_type=reinterpret
  Reinterpret1 : xbsIndex_r4/Reinterpret  force_arith_type=on  arith_type=Unsigned  force_bin_pt=on  bin_pt=0  block_type=reinterpret
  IQ : Outport
  wires:
  Reinterpret/1 -> Concat/1
  Reinterpret1/1 -> Concat/2
  I/1 -> Reinterpret/1
  Concat/1 -> IQ/1
  Q/1 -> Reinterpret1/1

== system wlan_phy_rx_pmd/CFO & Samp Buffer/FIFO/I/Q Slice ==
  IQ : Inport
  Reinterpret2 : xbsIndex_r4/Reinterpret  force_arith_type=on  arith_type="Signed  (2's comp)"  force_bin_pt=on  bin_pt=15  block_type=reinterpret
  Reinterpret3 : xbsIndex_r4/Reinterpret  force_arith_type=on  arith_type="Signed  (2's comp)"  force_bin_pt=on  bin_pt=15  block_type=reinterpret
  Slice : xbsIndex_r4/Slice  nbits=16  boolean_output=off  mode=Upper Bit Location + Width  bit1=0  base1=MSB of Input  bit0=0  base0=LSB of Input  dbl_ovrd=off  block_type=slice
  Slice1 : xbsIndex_r4/Slice  nbits=16  boolean_output=off  mode=Lower Bit Location + Width  bit1=0  base1=MSB of Input  bit0=0  base0=LSB of Input  dbl_ovrd=off  block_type=slice
  I : Outport
  Q : Outport  Port=2
  wires:
  Reinterpret3/1 -> Q/1
  Reinterpret2/1 -> I/1
  IQ/1 -> Slice/1, Slice1/1
  Slice1/1 -> Reinterpret3/1
  Slice/1 -> Reinterpret2/1

== system wlan_phy_rx_pmd/CFO & Samp Buffer/Samp Buffer/Concat ==
  I : Inport
  Q : Inport  Port=2
  Concat : xbsIndex_r4/Concat  num_inputs=2  dbl_ovrd=off  block_type=concat
  Reinterpret : xbsIndex_r4/Reinterpret  force_arith_type=on  arith_type=Unsigned  force_bin_pt=on  bin_pt=0  block_type=reinterpret
  Reinterpret1 : xbsIndex_r4/Reinterpret  force_arith_type=on  arith_type=Unsigned  force_bin_pt=on  bin_pt=0  block_type=reinterpret
  IQ : Outport
  wires:
  Reinterpret/1 -> Concat/1
  Reinterpret1/1 -> Concat/2
  I/1 -> Reinterpret/1
  Concat/1 -> IQ/1
  Q/1 -> Reinterpret1/1

== system wlan_phy_rx_pmd/CFO & Samp Buffer/Samp Buffer/FFT Load Ctrl ==
  Wr Addr : Inport
  data tready : Inport  Port=2
  LTS Sync : Inport  Port=3
  Skip Sel : Inport  Port=4
  FFT LTS Load : Inport  Port=5
  FFT Load : Inport  Port=6
  AddSub4 : xbsIndex_r4/AddSub  mode=Addition  use_carryin=off  use_carryout=off  en=off  latency=0  precision=User Defined  arith_type=Unsigned  n_bits=ceil(log2(preFFT_sampBuff_numSamps))  bin_pt=0  quantization=Truncate  overflow=Wrap  dbl_ovrd=off  use_behavioral_HDL=off  hw_selection=Fabric  pipelined=off  block_type=addsub
  Convert2 : xbsIndex_r4/Convert  gui_display_data_type=Boolean  arith_type=Boolean  n_bits=1  bin_pt=0  float_type=Single  exp_bits=8  fraction_bits=24  quantization=Truncate  overflow=Wrap  en=off  latency=0  dbl_ovrd=off  pipeline=off  block_type=convert
  Count-To Calc : Subsystem
  Ctrl : Scope  NumInputPorts=7
      settings: TimeRange=1322.580645161291  YMin=-1~70~59.85~166.25~-1~-1~166.25  YMax=1~135~66.15~183.75~1~1~183.75  SaveName=ScopeData5
  Delay : xbsIndex_r4/Delay  rst=off  infoeditControl=Selection of Reset will increase slice count due to use of real FFs and instead of SRLs  en=off  latency=1  dbl_ovrd=off  reg_retiming=off  block_type=delay
  First Samp Calc : Subsystem
  Gateway Out{1..7} (7 alike) : xbsIndex_r4/Gateway Out  inherit_from_input=off  hdl_port=off  timing_constraint=None  locs_specified=off  LOCs={}  UseAsDAC=off  DACChannel='1'  block_type=gatewayout
  Inverter : xbsIndex_r4/Inverter  en=off  latency=0  dbl_ovrd=off  block_type=inv
      settings: xl_area=[0 0 0 0 0 0 0]  block_version=VER_STRING_GOES_HERE
  Logical : xbsIndex_r4/Logical  logical_function=AND  inputs=2  en=off  latency=0  precision=Full  arith_type=Unsigned  n_bits=16  bin_pt=0  align_bp=on  dbl_ovrd=off  block_type=logical
  Logical1 : xbsIndex_r4/Logical  logical_function=OR  inputs=2  en=off  latency=0  precision=Full  arith_type=Unsigned  n_bits=16  bin_pt=0  align_bp=on  dbl_ovrd=off  block_type=logical
  Logical2 : xbsIndex_r4/Logical  logical_function=AND  inputs=2  en=off  latency=0  precision=Full  arith_type=Unsigned  n_bits=16  bin_pt=0  align_bp=on  dbl_ovrd=off  block_type=logical
  Logical3 : xbsIndex_r4/Logical  logical_function=OR  inputs=2  en=off  latency=0  precision=Full  arith_type=Unsigned  n_bits=16  bin_pt=0  align_bp=on  dbl_ovrd=off  block_type=logical
  Logical4 : xbsIndex_r4/Logical  logical_function=AND  inputs=3  en=off  latency=0  precision=Full  arith_type=Unsigned  n_bits=16  bin_pt=0  align_bp=on  dbl_ovrd=off  block_type=logical
  Posedge : Subsystem
  Rd Counter : xbsIndex_r4/Counter  cnt_type=Free Running  cnt_to=Inf  operation=Up  start_count=0  cnt_by_val=1  arith_type=Unsigned  n_bits=ceil(log2(preFFT_sampBuff_numSamps))  bin_pt=0  load_pin=on  rst=off  en=on  explicit_period=off  period=8  dbl_ovrd=off  use_behavioral_HDL=off  implementation=Fabric  block_type=counter
  Relational1 : xbsIndex_r4/Relational  mode=a=b  en=off  latency=0  dbl_ovrd=off  block_type=relational
  Relational2 : xbsIndex_r4/Relational  mode=a=b  en=off  latency=0  dbl_ovrd=off  block_type=relational
  Relational3 : xbsIndex_r4/Relational  mode=a<=b  en=off  latency=0  dbl_ovrd=off  block_type=relational
  Relational4 : xbsIndex_r4/Relational  mode=a>b  en=off  latency=0  dbl_ovrd=off  block_type=relational
  S-R Latch1 : Subsystem
  Samp Counter : xbsIndex_r4/Counter  cnt_type=Free Running  cnt_to=Inf  operation=Up  start_count=0  cnt_by_val=1  arith_type=Unsigned  n_bits=ceil(log2(MAX_NUM_SC+MAX_CP_LEN))  bin_pt=0  load_pin=off  rst=on  en=on  explicit_period=off  period=8  dbl_ovrd=off  use_behavioral_HDL=off  implementation=Fabric  block_type=counter
  Rd Addr : Outport
  Reading : Outport  Port=2
  FFT tvalid : Outport  Port=3
  FFT tlast : Outport  Port=4
  FFT Load Done : Outport  Port=5
  wires:
  Posedge/1(Q) -> FFT Load Done/1
  data tready/1 -> Convert2/1
  Wr Addr/1 -> First Samp Calc/1(Wr Addr), Relational1/1, Gateway Out2/1
  First Samp Calc/1(Samp Addr) -> Rd Counter/2, Gateway Out3/1
  Rd Counter/1 -> AddSub4/2, Rd Addr/1, Gateway Out6/1, Gateway Out4/1
  Skip Sel/1 -> Count-To Calc/1(Skip Sel)
  Count-To Calc/1(Samp+Skip) -> AddSub4/1, Relational3/1
  AddSub4/1 -> Relational1/2
  Logical4/1 -> Samp Counter/2, Logical1/2
  Convert2/1 -> Logical4/1
  FFT Load/1 -> Logical/1
  S-R Latch1/1(Q) -> Logical4/2, Logical2/1
  Relational1/1 -> Logical/2
  Samp Counter/1 -> Relational3/2, Relational4/2, Relational2/2, Gateway Out7/1
  Relational3/1 -> Samp Counter/1, Posedge/1(D), Inverter/1, S-R Latch1/2(R)
  Count-To Calc/2(Samp) -> Relational4/1, Relational2/1
  Relational4/1 -> Logical2/2
  Logical2/1 -> FFT tvalid/1, Gateway Out5/1
  Gateway Out5/1 -> Ctrl/6  "FFT tvalid"
  Gateway Out6/1 -> Ctrl/7  "Rd Addr"
  Gateway Out1/1 -> Ctrl/1  "LTS Sync"
  Gateway Out2/1 -> Ctrl/2  "Rx Samp Ind"
  Gateway Out3/1 -> Ctrl/3  "Init Samp Addr"
  Gateway Out4/1 -> Ctrl/4  "Rd Counter"
  Gateway Out7/1 -> Ctrl/5  "Samp Counter"
  Logical1/1 -> Rd Counter/3, Reading/1
  Delay/1 -> Rd Counter/1, Logical1/1
  Inverter/1 -> Logical4/3
  LTS Sync/1 -> First Samp Calc/2(LTS Sync), Delay/1, Gateway Out1/1
  Relational2/1 -> FFT tlast/1
  Logical/1 -> Logical3/1
  Logical3/1 -> S-R Latch1/1(S)
  FFT LTS Load/1 -> Logical3/2

== system wlan_phy_rx_pmd/CFO & Samp Buffer/Samp Buffer/FSM ==
  FFT Load Done : Inport
  LTS : Inport  Port=2
  Pkt Done : Inport  Port=3
  Convert : xbsIndex_r4/Convert  gui_display_data_type=Fixed-point  arith_type=Unsigned  n_bits=1  bin_pt=0  float_type=Single  exp_bits=8  fraction_bits=24  quantization=Truncate  overflow=Wrap  en=off  latency=0  dbl_ovrd=off  pipeline=off  block_type=convert
  Convert1 : xbsIndex_r4/Convert  gui_display_data_type=Fixed-point  arith_type=Unsigned  n_bits=1  bin_pt=0  float_type=Single  exp_bits=8  fraction_bits=24  quantization=Truncate  overflow=Wrap  en=off  latency=0  dbl_ovrd=off  pipeline=off  block_type=convert
  Convert2 : xbsIndex_r4/Convert  gui_display_data_type=Fixed-point  arith_type=Unsigned  n_bits=1  bin_pt=0  float_type=Single  exp_bits=8  fraction_bits=24  quantization=Truncate  overflow=Wrap  en=off  latency=0  dbl_ovrd=off  pipeline=off  block_type=convert
  Convert3 : xbsIndex_r4/Convert  gui_display_data_type=Boolean  arith_type=Boolean  n_bits=1  bin_pt=0  float_type=Single  exp_bits=8  fraction_bits=24  quantization=Truncate  overflow=Wrap  en=off  latency=0  dbl_ovrd=off  pipeline=off  block_type=convert
  Convert4 : xbsIndex_r4/Convert  gui_display_data_type=Boolean  arith_type=Boolean  n_bits=1  bin_pt=0  float_type=Single  exp_bits=8  fraction_bits=24  quantization=Truncate  overflow=Wrap  en=off  latency=0  dbl_ovrd=off  pipeline=off  block_type=convert
  FFT Load FSM : Scope  NumInputPorts=9
      settings: TimeRange=10000  YMin=-0.25~-0.25~0~0~0~-0.1~-0.1~-5~-5  YMax=0.2~0.2~300~300~4000~1.1~1.1~5~5  SaveName=ScopeData48
  Gateway Out{1..6} (6 alike) : xbsIndex_r4/Gateway Out  inherit_from_input=off  hdl_port=off  timing_constraint=None  locs_specified=off  LOCs={}  UseAsDAC=off  DACChannel='1'  block_type=gatewayout
  MCode : xbsIndex_r4/MCode  mfname=rx_fft_ctrl_fsm  explicit_period=on  period=1  inputsTable={'boundInpExpr'=>['','',''],'inputs'=>['lts_sync','fft_load_done','pkt_done']}  outputsTable={'outputs'=>['fft_load','samp_skip_mode'],'suppressOut'=>['off','off']}  dbl_ovrd=off  enable_stdout=off  enable_debug=off  suppress_output=on  defparams={}  hide_port_list={}  block_type=mcode
  FFT skip sel : Outport
  FFT LTS load : Outport  Port=2
  FFT Data load : Outport  Port=3
  wires:
  Pkt Done/1 -> Convert2/1
  Convert1/1 -> MCode/1, Gateway Out6/1
  MCode/1 -> Convert3/1
  MCode/2 -> Convert4/1
  Convert/1 -> MCode/2, Gateway Out5/1
  LTS/1 -> Convert1/1
  FFT Load Done/1 -> Convert/1
  Convert2/1 -> MCode/3, Gateway Out1/1
  MCode/3 -> FFT skip sel/1, Gateway Out4/1
  Convert3/1 -> FFT Data load/1, Gateway Out2/1
  Convert4/1 -> FFT LTS load/1, Gateway Out3/1
  Gateway Out5/1 -> FFT Load FSM/2  "FFT Load Done"
  Gateway Out6/1 -> FFT Load FSM/1  "LTS Sync"
  Gateway Out1/1 -> FFT Load FSM/3  "Pkt Done"
  Gateway Out2/1 -> FFT Load FSM/5  "FFT Data Load"
  Gateway Out3/1 -> FFT Load FSM/6  "FFT LTS Load"
  ? -> FFT Load FSM/4
  Gateway Out4/1 -> FFT Load FSM/7  "FFT skip sel"

== system wlan_phy_rx_pmd/CFO & Samp Buffer/Samp Buffer/Posedge ==
  note: Rising Edge Detection
  D : Inport
  Delay : xbsIndex_r4/Delay  rst=off  infoeditControl=Selection of Reset will increase slice count due to use of real FFs and instead of SRLs  en=off  latency=1  dbl_ovrd=off  reg_retiming=off  block_type=delay
      settings: infoedit=Hardware notes: A delay line is a chain, each link of which is an SRL16 followed by a flip-flop. If register retiming is enabled, the delay line is a chain of flip-flops.  xl_area=[1 1 0 0 0 0 0]  block_version=VER_STRING_GOES_HERE
  Inverter : xbsIndex_r4/Inverter  en=off  latency=0  dbl_ovrd=off  block_type=inv
      settings: xl_area=[0 0 0 0 0 0 0]  block_version=VER_STRING_GOES_HERE
  Logical1 : xbsIndex_r4/Logical  logical_function=AND  inputs=2  en=off  latency=0  precision=Full  arith_type=Unsigned  n_bits=8  bin_pt=2  align_bp=on  dbl_ovrd=off  block_type=logical
      settings: xl_area=[1 0 0 1 0 0 0]  block_version=VER_STRING_GOES_HERE
  Q : Outport
  wires:
  D/1 -> Logical1/2, Delay/1
  Logical1/1 -> Q/1
  Inverter/1 -> Logical1/1
  Delay/1 -> Inverter/1

== system wlan_phy_rx_pmd/CFO & Samp Buffer/Samp Buffer/Posedge3 ==
  note: Rising Edge Detection
  D : Inport
  Delay : xbsIndex_r4/Delay  rst=off  infoeditControl=Selection of Reset will increase slice count due to use of real FFs and instead of SRLs  en=off  latency=1  dbl_ovrd=off  reg_retiming=off  block_type=delay
      settings: infoedit=Hardware notes: A delay line is a chain, each link of which is an SRL16 followed by a flip-flop. If register retiming is enabled, the delay line is a chain of flip-flops.  xl_area=[1 1 0 0 0 0 0]  block_version=VER_STRING_GOES_HERE
  Inverter : xbsIndex_r4/Inverter  en=off  latency=0  dbl_ovrd=off  block_type=inv
      settings: xl_area=[0 0 0 0 0 0 0]  block_version=VER_STRING_GOES_HERE
  Logical1 : xbsIndex_r4/Logical  logical_function=AND  inputs=2  en=off  latency=0  precision=Full  arith_type=Unsigned  n_bits=8  bin_pt=2  align_bp=on  dbl_ovrd=off  block_type=logical
      settings: xl_area=[1 0 0 1 0 0 0]  block_version=VER_STRING_GOES_HERE
  Q : Outport
  wires:
  D/1 -> Logical1/2, Delay/1
  Logical1/1 -> Q/1
  Inverter/1 -> Logical1/1
  Delay/1 -> Inverter/1
